simulink model "TosAeb"  (R2021a)
settings: Solver=FixedStepDiscrete  StartTime=0.0  StopTime=inf  FixedStep=0.01  RelTol=1e-3
undefined symbols (not defined in the file or in its folder): ACCModSts, AccSts1, AEB_ObjectMovingOrientation, AEB_ObjectMovingState, CN_BTCActive, CN_CurrentGearR, CN_CurrentGearR_DHT, CN_PTCActive, CN_VDCActive, CN_VSC_EspOff, CN_VSC_FCW_AEB_Error, CN_VSC_FCW_AEB_NoDispay, CN_VSC_FCW_AEB_PerfmDgdtn, CN_VSC_FCW_AEB_TempUnAvlbl, CN_VSC_VehStandstill, CNaccelAvoidMax, CNaccelAvoidMin, CNbrakePedalPress, CNcomfortStrLeftAccelMax, CNcomfortStrLeftAccelMin, CNcomfortStrRightAccelMax, CNcomfortStrRightAccelMin, CNDrvDoorOpen, CNDrvModeEco, CNDrvModeNorm, CNDrvModeOther, CNDrvModeSport, CNDrvSBRreminder, CNNull, CNobjInvalidID, CNPassDoorOpen, CNPassSBRreminder, CNPEBSAccelNegInf, CNPEBSAccelNull, CNPEBSTimeInf, CNPEBSTimeNull, CNSTOPMAX, CNSTOPNULL, CNsubjWidth, CNSwtOFF, CNSwtON, CNTTCMAX, CNTTCNULL, CNVehConfigAEBCycNone, CNVehConfigAEBNone, CNVehConfigAEBPedNone, CNVehConfigFCWNone, CNVehConfigJANone, CollisionSeverity, FALSE, FCWSnvty, JACycObjCalcInfoDefaultParams, JAPedObjCalcInfoDefaultParams, JAVehObjCalcInfoDefaultParams, latCycObjCalcInfoDefaultParams, latPedObjCalcInfoDefaultParams, longObjCalcInfoDefaultParams, ModSts, movingStatus, ObjectType ... (+1112)
libraries: simulink x3031, AEBSFunctionLib x50, Ta_Library x33, AEBSStateMachineLib x23, Mylibrary x12, simulink_extras x7, ObjSelect_lib x3, dspstat3 x2
(part 6 of 102)

== system TosAeb/AEB_Objsel/TA_Processor/AEB_ObjSelect/TargetInfoCheck/Each_Set ==
  TA_ObjSumInfo : Inport
  Bus Selector : BusSelector  OutputSignals=TA_ObjID,TA_ObjType,TA_ObjState,TA_ObjOrientation,TA_ObjfusType,TA_ObjLaneAssign,TA_ObjDx,TA_ObjDy,TA_ObjDxStd,TA_ObjDyStd,TA_ObjVx,TA_ObjVy,TA_ObjVxStd,TA_ObjVyStd,TA_ObjVxAbs,TA_ObjVyAbs,TA_ObjLength,TA_ObjWidth,TA_ObjHight,TA_ObjAx,TA_ObjAy,TA_ObjAxAbs,TA_ObjAyAbs,TA_ObjConfidence,TA_ObjCenterAngle,TA_ObjHeadAngle,TA_ObjHeadAngleStd,TA_ObjID
  Signal Conversion{,1..27} (28 alike) : SignalConversion  OverrideOpt=off
  TA_ObjID : Outport
  TA_ObjType : Outport  Port=2
  TA_ObjState : Outport  Port=3
  TA_ObjOrientation : Outport  Port=4
  TA_ObjfusType : Outport  Port=5
  TA_ObjLaneAssign : Outport  Port=6
  TA_ObjDx : Outport  Port=7
  TA_ObjDy : Outport  Port=8
  TA_ObjDxStd : Outport  Port=9
  TA_ObjDyStd : Outport  Port=10
  TA_ObjVx : Outport  Port=11
  TA_ObjVy : Outport  Port=12
  TA_ObjVxStd : Outport  Port=13
  TA_ObjVyStd : Outport  Port=14
  TA_ObjVxAbs : Outport  Port=15
  TA_ObjVyAbs : Outport  Port=16
  TA_ObjLength : Outport  Port=17
  TA_ObjWidth : Outport  Port=18
  TA_ObjHight : Outport  Port=19
  TA_ObjAx : Outport  Port=20
  TA_ObjAy : Outport  Port=21
  TA_ObjAxAbs : Outport  Port=22
  TA_ObjAyAbs : Outport  Port=23
  TA_ObjConfidence : Outport  Port=24
  TA_ObjCenterAngle : Outport  Port=25
  TA_ObjHeadAngle : Outport  Port=26
  TA_ObjHeadAngleStd : Outport  Port=27
  IDForeach : Outport  Port=28
  wires:
  TA_ObjSumInfo/1 -> Bus Selector/1
  Bus Selector/1 -> Signal Conversion/1  "<TA_ObjID>"
  Bus Selector/2 -> Signal Conversion1/1  "<TA_ObjType>"
  Bus Selector/3 -> Signal Conversion2/1  "<TA_ObjState>"
  Bus Selector/4 -> Signal Conversion3/1  "<TA_ObjOrientation>"
  Bus Selector/5 -> Signal Conversion4/1  "<TA_ObjfusType>"
  Bus Selector/6 -> Signal Conversion5/1  "<TA_ObjLaneAssign>"
  Bus Selector/7 -> Signal Conversion6/1  "<TA_ObjDx>"
  Bus Selector/8 -> Signal Conversion7/1  "<TA_ObjDy>"
  Bus Selector/9 -> Signal Conversion8/1  "<TA_ObjDxStd>"
  Bus Selector/10 -> Signal Conversion9/1  "<TA_ObjDyStd>"
  Bus Selector/11 -> Signal Conversion10/1  "<TA_ObjVx>"
  Bus Selector/12 -> Signal Conversion11/1  "<TA_ObjVy>"
  Bus Selector/13 -> Signal Conversion12/1  "<TA_ObjVxStd>"
  Bus Selector/14 -> Signal Conversion13/1  "<TA_ObjVyStd>"
  Bus Selector/15 -> Signal Conversion14/1  "<TA_ObjVxAbs>"
  Bus Selector/16 -> Signal Conversion15/1  "<TA_ObjVyAbs>"
  Bus Selector/17 -> Signal Conversion16/1  "<TA_ObjLength>"
  Bus Selector/18 -> Signal Conversion17/1  "<TA_ObjWidth>"
  Bus Selector/19 -> Signal Conversion18/1  "<TA_ObjHight>"
  Bus Selector/20 -> Signal Conversion19/1  "<TA_ObjAx>"
  Bus Selector/21 -> Signal Conversion20/1  "<TA_ObjAy>"
  Bus Selector/22 -> Signal Conversion21/1  "<TA_ObjAxAbs>"
  Bus Selector/23 -> Signal Conversion22/1  "<TA_ObjAyAbs>"
  Bus Selector/24 -> Signal Conversion23/1  "<TA_ObjConfidence>"
  Bus Selector/25 -> Signal Conversion24/1  "<TA_ObjCenterAngle>"
  Bus Selector/26 -> Signal Conversion25/1  "<TA_ObjHeadAngle>"
  Bus Selector/27 -> Signal Conversion26/1  "<TA_ObjHeadAngleStd>"
  Bus Selector/28 -> Signal Conversion27/1  "<TA_ObjID>"
  Signal Conversion/1 -> TA_ObjID/1
  Signal Conversion1/1 -> TA_ObjType/1
  Signal Conversion2/1 -> TA_ObjState/1
  Signal Conversion3/1 -> TA_ObjOrientation/1
  Signal Conversion4/1 -> TA_ObjfusType/1
  Signal Conversion5/1 -> TA_ObjLaneAssign/1
  Signal Conversion6/1 -> TA_ObjDx/1
  Signal Conversion7/1 -> TA_ObjDy/1
  Signal Conversion8/1 -> TA_ObjDxStd/1
  Signal Conversion9/1 -> TA_ObjDyStd/1
  Signal Conversion10/1 -> TA_ObjVx/1
  Signal Conversion11/1 -> TA_ObjVy/1
  Signal Conversion12/1 -> TA_ObjVxStd/1
  Signal Conversion13/1 -> TA_ObjVyStd/1
  Signal Conversion14/1 -> TA_ObjVxAbs/1
  Signal Conversion15/1 -> TA_ObjVyAbs/1
  Signal Conversion16/1 -> TA_ObjLength/1
  Signal Conversion17/1 -> TA_ObjWidth/1
  Signal Conversion18/1 -> TA_ObjHight/1
  Signal Conversion19/1 -> TA_ObjAx/1
  Signal Conversion20/1 -> TA_ObjAy/1
  Signal Conversion21/1 -> TA_ObjAxAbs/1
  Signal Conversion22/1 -> TA_ObjAyAbs/1
  Signal Conversion23/1 -> TA_ObjConfidence/1
  Signal Conversion24/1 -> TA_ObjCenterAngle/1
  Signal Conversion25/1 -> TA_ObjHeadAngle/1
  Signal Conversion26/1 -> TA_ObjHeadAngleStd/1
  Signal Conversion27/1 -> IDForeach/1

== system TosAeb/AEB_Objsel/TA_Processor/AEB_ObjSelect/TargetSelect/JA_ObjSelect ==
  TA_ObjInfo : Inport
  JAObjCollOut : Inport  Port=2
  Bus Selector : BusSelector  OutputSignals=TA_ObjID
  Bus Selector1 : BusSelector  OutputSignals=JA_ObjTcc,JA_ObjCollFlg
  MATLAB Function : MATLAB Function  TreatAsAtomicUnit=on
  JA_ObjSortNum : Outport
  JA_VaildID : Outport  Port=2
  wires:
  TA_ObjInfo/1 -> Bus Selector/1
  JAObjCollOut/1 -> Bus Selector1/1
  Bus Selector/1 -> MATLAB Function/1(ID)  "<TA_ObjID>"
  Bus Selector1/1 -> MATLAB Function/2(TTC)  "<JA_ObjTcc>"
  Bus Selector1/2 -> MATLAB Function/3(CllsnFlg)  "<JA_ObjCollFlg>"
  MATLAB Function/1(SortNum) -> JA_ObjSortNum/1
  MATLAB Function/2(VaildID) -> JA_VaildID/1

== system TosAeb/AEB_Objsel/TA_Processor/AEB_ObjSelect/TargetSelect/Lat_ObjSelect ==
  LatObj_Valid : Inport
  Lat_ObjIntersect : Inport  Port=2
  Lat_TTR : Inport  Port=3
  TA_ObjInfo : Inport  Port=4
  AEB_LatObj : Subsystem
  Lat_ValidNumber : Subsystem  TreatAsAtomicUnit=on
  Lat_ObjSortNum : Outport
  Lat_ObjID : Outport  Port=2
  wires:
  Lat_ValidNumber/1(Lat_ObjNum) -> AEB_LatObj/1(Lat_ObjNum)
  TA_ObjInfo/1 -> AEB_LatObj/3(TA_ObjInfo)
  LatObj_Valid/1 -> AEB_LatObj/2(LatObj_Valid), Lat_ValidNumber/1(LatObj_Valid)
  Lat_ObjIntersect/1 -> AEB_LatObj/4(Lat_ObjIntersect)
  Lat_TTR/1 -> AEB_LatObj/5(Lat_TTR)
  AEB_LatObj/1(Lat_ObjSortNum) -> Lat_ObjSortNum/1
  AEB_LatObj/2(Lat_ObjID) -> Lat_ObjID/1

== system TosAeb/AEB_Objsel/TA_Processor/AEB_ObjSelect/TargetSelect/Long_ObjSelect ==
  TA_ObjInfo : Inport
  VehSelfData : Inport  Port=2
  Boudingbox_Calculate : Inport  Port=3
  Obj_Coef : Inport  Port=4
  TTI : Inport  Port=5
  AccTgtIdn : Inport  Port=6
  Overlap_Rate : Inport  Port=7
  Obj_IDConfirm : Subsystem
  Long_ObjSortNum : Outport
  Long_ObjID : Outport  Port=2
  long_TTC : Outport  Port=3
  wires:
  VehSelfData/1 -> Obj_IDConfirm/2(VehSelfData)
  Obj_Coef/1 -> Obj_IDConfirm/4(Obj_Coef)
  TA_ObjInfo/1 -> Obj_IDConfirm/1(TA_ObjInfo)
  Boudingbox_Calculate/1 -> Obj_IDConfirm/3(Boudingbox_Calculate)
  TTI/1 -> Obj_IDConfirm/5(TTI)
  AccTgtIdn/1 -> Obj_IDConfirm/6(AccTgtIdn)
  Obj_IDConfirm/1(ValidTarget_ID) -> Long_ObjID/1
  Obj_IDConfirm/2(ID_num) -> Long_ObjSortNum/1
  Overlap_Rate/1 -> Obj_IDConfirm/7(Overlap_Rate)
  Obj_IDConfirm/3(long_TTC) -> long_TTC/1

== system TosAeb/AEB_Objsel/TA_Processor/PreProcessor/ExtractAccTargetIdIfAccActive/ConfirmAccId ==
  Acc_TaP0Id : Inport
  Constant : Constant  Value=uint8(0)  OutDataTypeStr=uint8
  Constant1 : Constant  Value=P_TA_AccTarCfmDlyInTaSlection
  Constant2 : Constant  Value=P_TA_AccTarCfmDlyEnadInTaSlection
  Constant4 : Constant  Value=P_TA_CallFrequencyInTaSlection
  Logical Operator : Logic  AllPortsSameDT=off  OutDataTypeStr=boolean
  OnDelay : Ta_Library/OnDelay  SystemSampleTime=-1  VariantActivationTime=update diagram  Latency=0  AutoFrameSizeCalculation=off
  Relational Operator : RelationalOperator  Operator===  OutDataTypeStr=boolean  RndMeth=Simplest
  Switch : Switch  Criteria=u2 ~= 0
  Unit Delay : UnitDelay  SampleTime=-1  HasFrameUpgradeWarning=on
  Confirmed_ AccP0Id : Outport
  wires:
  Constant2/1 -> Logical Operator/2
  Constant1/1 -> OnDelay/2
  Constant4/1 -> OnDelay/3
  Logical Operator/1 -> Switch/2
  Switch/1 -> Confirmed_ AccP0Id/1
  Constant/1 -> Switch/3
  OnDelay/1 -> Logical Operator/1  "AccIdHasBeenConstantForLongTime"
  Unit Delay/1 -> Relational Operator/2
  Relational Operator/1 -> OnDelay/1  "SameIdInLastSample"
  Acc_TaP0Id/1 -> Unit Delay/1, Relational Operator/1, Switch/1

== system TosAeb/AEB_Objsel/TA_Processor/PreProcessor/ExtractAccTargetIdIfAccActive/Enumerated Constant3 ==
  Constant : Constant  DialogController=Simulink.DDGSource  Value=AccSts1.Stop  OutDataTypeStr=Enum: AccSts1  LockScale=on
  Out : Outport
  wires:
  Constant/1 -> Out/1

== system TosAeb/AEB_Objsel/TA_Processor/PreProcessor/ExtractAccTargetIdIfAccActive/Enumerated Constant4 ==
  Constant : Constant  DialogController=Simulink.DDGSource  Value=AccSts1.StopTmp  OutDataTypeStr=Enum: AccSts1  LockScale=on
  Out : Outport
  wires:
  Constant/1 -> Out/1

== system TosAeb/AEB_Objsel/TA_Processor/PreProcessor/ExtractAccTargetIdIfAccActive/Enumerated Constant5 ==
  Constant : Constant  DialogController=Simulink.DDGSource  Value=AccSts1.Actv  OutDataTypeStr=Enum: AccSts1  LockScale=on
  Out : Outport
  wires:
  Constant/1 -> Out/1

== system TosAeb/AEB_Objsel/TA_Processor/PreProcessor/ObjectPrecessor/TAObjInfoCalc ==
  VehSelfIn : Inport
  Fus_TargetBus : Inport  Port=2
  Bus Selector : BusSelector  OutputSignals=Cam_ObsID_u8,Cam_ObsValid_u8,Cam_ObsAge_u16,Cam_ObsConfidence_u8,Cam_ObsMotionCategory_u8,Cam_ObsMotionStatus_u8,Cam_ObsMeasureStatus_u8,Cam_ObsClass_u8,Cam_ObsVhSubType_u8,Cam_ObsPedSubType_u8,Cam_ObsPedPos_u8,Cam_ObsPedOrientation_u8,Cam_ObsLaneAssign_u8,Cam_ObsLength_s,Cam_ObsWidth_s,Cam_ObsHeight_s,Cam_ObsCIPV_b,Cam_ObsMCP_b,Cam_ObsBlinker_u8,Cam_ObsBrkLgt_b,Cam_ObsCenterAngle_s,Cam_ObsPosType_u8,Cam_ObsPosX_s,Cam_ObsPosY_s,Cam_ObsVelX_s,Cam_ObsVelY_s,Cam_ObsAccX_s,Cam_ObsPosXSTD_s,Cam_ObsPosYSTD_s,Cam_ObsVelXSTD_s,Cam_ObsVelYSTD_s,Cam_ObsAccY_s,Cam_ObsAngle_s,Cam_ObsAngleSTD_s,Cam_ObsVelXAbs_s,Cam_ObsVelYAbs_s,Cam_ObsCornerPntX_s,Cam_ObsCutInDis_s,Cam_ObsCornerPntY_s,Cam_ObsCutInFlg_u8,Cam_ObsCutInSpd_s,Cam_ObsCutInSpdValid_b,Cam_ObsProNotOnRoad_b,Cam_ObsProCoverdByOthers_b,Cam_ObsProObserveJump_b,Cam_ObsProObserveSide_b,Cam_ObsProBtTapLevel1_b,Cam_ObsProBtTapLevel2_b,Cam_ObsProBtTapLevel3_b,Cam_ObsID_u8
  For Each Subsystem : Subsystem  TreatAsAtomicUnit=on
  Signal_Assignment : Subsystem
  TA_ObjInfo : Outport
  wires:
  For Each Subsystem/10(Fus_ObjQuality) -> Signal_Assignment/10(Fus_ObjQuality)
  For Each Subsystem/5(Fus_ObjLaneAssign) -> Signal_Assignment/5(Fus_ObjLaneAssign)
  For Each Subsystem/3(Fus_ObjState) -> Signal_Assignment/3(Fus_ObjState)
  For Each Subsystem/9(Fus_ObjVy) -> Signal_Assignment/9(Fus_ObjVy)
  For Each Subsystem/23(Fus_VelySTD) -> Signal_Assignment/23(Fus_VelySTD)
  For Each Subsystem/1(Fus_ObjID) -> Signal_Assignment/1(Fus_ObjID)
  For Each Subsystem/6(Fus_ObjDy) -> Signal_Assignment/6(Fus_ObjDy)
  For Each Subsystem/14(Fus_ObjVyAbs) -> Signal_Assignment/14(Fus_ObjVyAbs)
  For Each Subsystem/17(Fus_ObjLength) -> Signal_Assignment/17(Fus_ObjLength)
  For Each Subsystem/8(Fus_ObjVx) -> Signal_Assignment/8(Fus_ObjVx)
  For Each Subsystem/7(Fus_ObjDx) -> Signal_Assignment/7(Fus_ObjDx)
  For Each Subsystem/18(Fus_ObjWidth) -> Signal_Assignment/18(Fus_ObjWidth)
  For Each Subsystem/27(Fus_AyAbs) -> Signal_Assignment/27(Fus_AyAbs)
  For Each Subsystem/11(Fus_ObjAy) -> Signal_Assignment/11(Fus_ObjAy)
  For Each Subsystem/12(Fus_ObjAx) -> Signal_Assignment/12(Fus_ObjAx)
  For Each Subsystem/20(Fus_dxSTD) -> Signal_Assignment/20(Fus_dxSTD)
  For Each Subsystem/21(Fus_dySTD) -> Signal_Assignment/21(Fus_dySTD)
  Signal_Assignment/1(TA_ObjInfo) -> TA_ObjInfo/1
  For Each Subsystem/19(Fus_ObjHight) -> Signal_Assignment/19(Fus_ObjHight)
  For Each Subsystem/22(Fus_VelxSTD) -> Signal_Assignment/22(Fus_VelxSTD)
  For Each Subsystem/16(Fus_ObjType) -> Signal_Assignment/16(Fus_ObjType)
  For Each Subsystem/13(Fus_ObjVxAbs) -> Signal_Assignment/13(Fus_ObjVxAbs)
  For Each Subsystem/2(Fus_ObjOrientation) -> Signal_Assignment/2(Fus_ObjOrientation)
  For Each Subsystem/26(Fus_AxAbs) -> Signal_Assignment/26(Fus_AxAbs)
  For Each Subsystem/4(Fus_ObjfusType) -> Signal_Assignment/4(Fus_ObjfusType)
  For Each Subsystem/15(Fus_ObjHeadAngle) -> Signal_Assignment/15(Fus_ObjHeadAngle)
  VehSelfIn/1 -> For Each Subsystem/51(VehSelfIn)
  For Each Subsystem/25(Fus_CenterAngle) -> Signal_Assignment/25(Fus_CenterAngle)
  Fus_TargetBus/1 -> Bus Selector/1
  For Each Subsystem/24(Fus_HeadAngleSTD) -> Signal_Assignment/24(Fus_HeadAngleSTD)
  Bus Selector/1 -> For Each Subsystem/1(Cam_ObsID_u8)  "<Cam_ObsID_u8>"
  Bus Selector/2 -> For Each Subsystem/2(Cam_ObsValid_u8)  "<Cam_ObsValid_u8>"
  Bus Selector/3 -> For Each Subsystem/3(Cam_ObsAge_u16)  "<Cam_ObsAge_u16>"
  Bus Selector/4 -> For Each Subsystem/4(Cam_ObsConfidence_u8)  "<Cam_ObsConfidence_u8>"
  Bus Selector/5 -> For Each Subsystem/5(Cam_ObsMotionCategory_u8)  "<Cam_ObsMotionCategory_u8>"
  Bus Selector/6 -> For Each Subsystem/6(Cam_ObsMotionStatus_u8)  "<Cam_ObsMotionStatus_u8>"
  Bus Selector/7 -> For Each Subsystem/7(Cam_ObsMeasureStatus_u8)  "<Cam_ObsMeasureStatus_u8>"
  Bus Selector/8 -> For Each Subsystem/8(Cam_ObsClass_u8)  "<Cam_ObsClass_u8>"
  Bus Selector/9 -> For Each Subsystem/9(Cam_ObsVhSubType_u8)  "<Cam_ObsVhSubType_u8>"
  Bus Selector/10 -> For Each Subsystem/10(Cam_ObsPedSubType_u8)  "<Cam_ObsPedSubType_u8>"
  Bus Selector/11 -> For Each Subsystem/11(Cam_ObsPedPos_u8)  "<Cam_ObsPedPos_u8>"
  Bus Selector/12 -> For Each Subsystem/12(Cam_ObsPedOrientation_u8)  "<Cam_ObsPedOrientation_u8>"
  Bus Selector/13 -> For Each Subsystem/13(Cam_ObsLaneAssign_u8)  "<Cam_ObsLaneAssign_u8>"
  Bus Selector/14 -> For Each Subsystem/14(Cam_ObsLength_s)  "<Cam_ObsLength_s>"
  Bus Selector/15 -> For Each Subsystem/15(Cam_ObsWidth_s)  "<Cam_ObsWidth_s>"
  Bus Selector/16 -> For Each Subsystem/16(Cam_ObsHeight_s)  "<Cam_ObsHeight_s>"
  Bus Selector/17 -> For Each Subsystem/17(Cam_ObsCIPV_b)  "<Cam_ObsCIPV_b>"
  Bus Selector/18 -> For Each Subsystem/18(Cam_ObsMCP_b)  "<Cam_ObsMCP_b>"
  Bus Selector/19 -> For Each Subsystem/19(Cam_ObsBlinker_u8)  "<Cam_ObsBlinker_u8>"
  Bus Selector/20 -> For Each Subsystem/20(Cam_ObsBrkLgt_b)  "<Cam_ObsBrkLgt_b>"
  Bus Selector/21 -> For Each Subsystem/21(Cam_ObsCenterAngle_s)  "<Cam_ObsCenterAngle_s>"
  Bus Selector/22 -> For Each Subsystem/22(Cam_ObsPosType_u8)  "<Cam_ObsPosType_u8>"
  Bus Selector/23 -> For Each Subsystem/23(Cam_ObsPosX_s)  "<Cam_ObsPosX_s>"
  Bus Selector/24 -> For Each Subsystem/24(Cam_ObsPosY_s)  "<Cam_ObsPosY_s>"
  Bus Selector/25 -> For Each Subsystem/25(Cam_ObsVelX_s)  "<Cam_ObsVelX_s>"
  Bus Selector/26 -> For Each Subsystem/26(Cam_ObsVelY_s)  "<Cam_ObsVelY_s>"
  Bus Selector/27 -> For Each Subsystem/27(Cam_ObsAccX_s)  "<Cam_ObsAccX_s>"
  Bus Selector/28 -> For Each Subsystem/28(Cam_ObsPosXSTD_s)  "<Cam_ObsPosXSTD_s>"
  Bus Selector/29 -> For Each Subsystem/29(Cam_ObsPosYSTD_s)  "<Cam_ObsPosYSTD_s>"
  Bus Selector/30 -> For Each Subsystem/30(Cam_ObsVelXSTD_s)  "<Cam_ObsVelXSTD_s>"
  Bus Selector/31 -> For Each Subsystem/31(Cam_ObsVelYSTD_s)  "<Cam_ObsVelYSTD_s>"
  Bus Selector/32 -> For Each Subsystem/32(Cam_ObsAccY_s)  "<Cam_ObsAccY_s>"
  Bus Selector/33 -> For Each Subsystem/33(Cam_ObsAngle_s)  "<Cam_ObsAngle_s>"
  Bus Selector/34 -> For Each Subsystem/34(Cam_ObsAngleSTD_s)  "<Cam_ObsAngleSTD_s>"
  Bus Selector/35 -> For Each Subsystem/35(Cam_ObsVelXAbs_s)  "<Cam_ObsVelXAbs_s>"
  Bus Selector/36 -> For Each Subsystem/36(Cam_ObsVelYAbs_s)  "<Cam_ObsVelYAbs_s>"
  Bus Selector/37 -> For Each Subsystem/37(Cam_ObsCornerPntX_s)  "<Cam_ObsCornerPntX_s>"
  Bus Selector/38 -> For Each Subsystem/38(Cam_ObsCutInDis_s)  "<Cam_ObsCutInDis_s>"
  Bus Selector/39 -> For Each Subsystem/39(Cam_ObsCornerPntY_s)  "<Cam_ObsCornerPntY_s>"
  Bus Selector/40 -> For Each Subsystem/40(Cam_ObsCutInFlg_u8)  "<Cam_ObsCutInFlg_u8>"
  Bus Selector/41 -> For Each Subsystem/41(Cam_ObsCutInSpd_s)  "<Cam_ObsCutInSpd_s>"
  Bus Selector/42 -> For Each Subsystem/42(Cam_ObsCutInSpdValid_b)  "<Cam_ObsCutInSpdValid_b>"
  Bus Selector/43 -> For Each Subsystem/43(Cam_ObsProNotOnRoad_b)  "<Cam_ObsProNotOnRoad_b>"
  Bus Selector/44 -> For Each Subsystem/44(Cam_ObsProCoverdByOthers_b)  "<Cam_ObsProCoverdByOthers_b>"
  Bus Selector/45 -> For Each Subsystem/45(Cam_ObsProObserveJump_b)  "<Cam_ObsProObserveJump_b>"
  Bus Selector/46 -> For Each Subsystem/46(Cam_ObsProObserveSide_b)  "<Cam_ObsProObserveSide_b>"
  Bus Selector/47 -> For Each Subsystem/47(Cam_ObsProBtTapLevel1_b)  "<Cam_ObsProBtTapLevel1_b>"
  Bus Selector/48 -> For Each Subsystem/48(Cam_ObsProBtTapLevel2_b)  "<Cam_ObsProBtTapLevel2_b>"
  Bus Selector/49 -> For Each Subsystem/49(Cam_ObsProBtTapLevel3_b)  "<Cam_ObsProBtTapLevel3_b>"
  Bus Selector/50 -> For Each Subsystem/50(IDForEach)  "<Cam_ObsID_u8>"

== system TosAeb/AEB_Objsel/TA_Processor/PreProcessor/VehiclePreProcessor/CalculateCurvature ==
  note: [8 10]
  note: [0 1]
  note: 自车纵向车速低于8m/s时，使用YawRate来计算自车运动曲率
      | 自车纵向车速高于10m/s时，使用二自由度自行车模型来计算自车运动曲率
      | 自车纵向车速在8-10m/s范围内时，采用插值取权重的方法来计算自车运动曲率
  VehSelf : Inport
  Bus Selector1 : BusSelector  OutputSignals=ABS_VehicleSpeed,SAS_SteeringWheelAngle
  Bus Selector2 : BusSelector  OutputSignals=SAS_SteeringWheelSpeed
  Bus Selector4 : BusSelector  OutputSignals=YawRate
  Constant : Constant  Value=single(1)
  Constant1 : Constant  Value=single(0)
  LookUpWeightFactor : Lookup_n-D  NumberOfTableDimensions=1  BreakpointsForDimension1=P_DM_DrvVLgtForCrvtTable  Table=P_DM_DrvCrvtCalcWeightFactor  ExtrapMethod=Clip  RemoveProtectionInput=on  UseLastTableValue=on  InternalRulePriority=Speed  RndMeth=Simplest  InputSameDT=on
      settings: AttributesFormatString=|
      | UseLastTableValue=%<UseLastTableValue>
      | RemoveProtectionInput=%<RemoveProtectionInput>
      | RndMeth=%<RndMeth>
  LowPassFilter : Subsystem
  SafeDivide : Subsystem  TreatAsAtomicUnit=on  mask(Safe divide){DataType=single, DataTypeMax=0, DataTypeMin=0}
      mask prompts: DataType "Data type:"
      mask help:
      | This block offers division protected from division by zero.
      |
      | When division by zero is detected, a very large number will be returned with the same sign as the numerator.
      |
      | In the case zero divided by zero, the output has been defined as zero.
      |
      | Both floating-point numbers and integers are supported.
      mask init:
      | % Author: Jakob Andersson (jande573)
      | 
      | % Test if the selected data type is a floating point type or an integer
      | % The supported data types is selected on the parameters pane
      | if(strcmp(DataType,'double')||strcmp(DataType,'single'))
      |     % Find the max and min values of the selected data type
      |     DataTypeMax=fix(sqrt(realmax(DataType))); % In order to avoid errors when operating on the output
      |     DataTypeMin=-fix(sqrt(realmax(DataType))); % In order to avoid errors when operating on the output
      | else
      |     % Find the max and min values of the selected data type
      |     DataTypeMax=intmax(DataType);
      |     DataTypeMin=-intmax(DataType);
      | end
      settings: RTWSystemCode=Inline
      mask Help:
      | This block offers division protected from division by zero.
      | 
      | When division by zero is detected, a very large number will be returned with the same sign as the numerator.
      | 
      | In the case zero divided by zero, the output has been defined as zero.
      | 
      | Both floating-point numbers and integers are supported.
      mask Display:
      | % Author: Jakob Andersson (jande573)
      | 
      | % Display the allowed data type
      | disp(DataType);
      | 
      | % Display the port symbols
      | port_label('input',1,'\bullet','texmode','on');
      | port_label('input',2,'\div','texmode','on');
  SafeDivide1 : Subsystem  TreatAsAtomicUnit=on  mask(Safe divide){DataType=single, DataTypeMax=0, DataTypeMin=0}
      mask prompts: DataType "Data type:"
      mask help:
      | This block offers division protected from division by zero.
      |
      | When division by zero is detected, a very large number will be returned with the same sign as the numerator.
      |
      | In the case zero divided by zero, the output has been defined as zero.
      |
      | Both floating-point numbers and integers are supported.
      mask init: same as TosAeb/AEB_Objsel/TA_Processor/PreProcessor/VehiclePreProcessor/CalculateCurvature/SafeDivide
      settings: RTWSystemCode=Inline
      mask Help:
      | This block offers division protected from division by zero.
      | 
      | When division by zero is detected, a very large number will be returned with the same sign as the numerator.
      | 
      | In the case zero divided by zero, the output has been defined as zero.
      | 
      | Both floating-point numbers and integers are supported.
      mask Display:
      | % Author: Jakob Andersson (jande573)
      | 
      | % Display the allowed data type
      | disp(DataType);
      | 
      | % Display the port symbols
      | port_label('input',1,'\bullet','texmode','on');
      | port_label('input',2,'\div','texmode','on');
  Saturation1 : Saturate  UpperLimit=single(0.1)  LowerLimit=single(-0.1)
      settings: AttributesFormatString=|
      | Limits:
      | [%<LowerLimit>, %<UpperLimit>]
  Saturation2 : Saturate  UpperLimit=single(0.1)  LowerLimit=single(-0.1)
      settings: AttributesFormatString=|
      | Limits:
      | [%<LowerLimit>, %<UpperLimit>]
  StaticBicycleModelC0 : Subsystem
  StaticBicycleModelC1 : Subsystem
  Weighting : Subsystem  mask(Blending){}
      mask help:
      | Mixes input X1 with input X2 using the weight factor, w.
      | Y = X1*w + X2*(1-w).
      | If w >= 1, Y = X1.
      | If w <= 0, Y = X2.
  Weighting1 : Subsystem  mask(Blending){}
      mask help:
      | Mixes input X1 with input X2 using the weight factor, w.
      | Y = X1*w + X2*(1-w).
      | If w >= 1, Y = X1.
      | If w <= 0, Y = X2.
  Curvature : Outport
  CurvatureRate : Outport  Port=2
  CurvatureRadius : Outport  Port=3
  wires:
  Saturation2/1 -> CurvatureRate/1  "CurvatureRate"
  Saturation1/1 -> Curvature/1, SafeDivide1/2(Denominator)  "Curvature"
  Weighting1/1(Y) -> Saturation2/1
  [Weight] -> Weighting1/2(WeightFactor)
  Constant1/1 -> Weighting1/1(X1)
  LowPassFilter/1(PinionAngleRate) -> StaticBicycleModelC1/2(PinionSteerAgRate)
  SafeDivide1/1(Quotient) -> CurvatureRadius/1  "CurvatureRadius"
  Constant/1 -> SafeDivide1/1(Numerator)
  Bus Selector1/1 -> LookUpWeightFactor/1, SafeDivide/2(Denominator), StaticBicycleModelC0/1(VLgt), [VLgt]  "<ABS_VehicleSpeed>"
  StaticBicycleModelC0/1(CurvatureRear) -> Weighting/3(X2)  "PinionAngleBasedCurvature"
  Bus Selector4/1 -> SafeDivide/1(Numerator)  "<YawRate>"
  SafeDivide/1(Quotient) -> Weighting/1(X1)  "YawRateBasedCurvature"
  LookUpWeightFactor/1 -> Weighting/2(WeightFactor), [Weight]  "WeightFactor"
  Weighting/1(Y) -> Saturation1/1  "Curvature"
  StaticBicycleModelC1/1(CurvatureRear) -> Weighting1/3(X2)  "PinionAngleBasedCurvatureRate"
  [VLgt] -> StaticBicycleModelC1/1(VLgt)
  VehSelf/1 -> Bus Selector2/1, Bus Selector1/1, Bus Selector4/1
  Bus Selector1/2 -> StaticBicycleModelC0/2(PinionSteerAg)  "<SAS_SteeringWheelAngle>"
  Bus Selector2/1 -> LowPassFilter/1(PinionAngleRate_in)  "<SAS_SteeringWheelSpeed>"

== system TosAeb/AEB_Objsel/TA_Processor/PreProcessor/VehiclePreProcessor/LongitudinalAccelerationWithoutCMbB ==
  note: CMbB激活下发DriverAcceleration
      |           未激活下发ALgt
  VehSelf : Inport
  ActtnDataFromCMbB : Inport  Port=2
  Bus Selector1 : BusSelector  OutputSignals=ABS_MasterCylinderPressure
  Bus Selector2 : BusSelector  OutputSignals=AX1
  Constant : Constant  OutDataTypeStr=boolean
  Relop_1 : RelationalOperator  Operator===  OutDataTypeStr=boolean  RndMeth=Nearest
  Switch : Switch  Criteria=u2 ~= 0
  Unary Minus : UnaryMinus
  DrvrALgt : Outport
  wires:
  Constant/1 -> Relop_1/2
  Relop_1/1 -> Switch/2  "CMbBActive"
  Switch/1 -> DrvrALgt/1  "DrvrALgt"
  ActtnDataFromCMbB/1 -> Relop_1/1
  VehSelf/1 -> Bus Selector2/1, Bus Selector1/1
  Bus Selector1/1 -> Switch/3  "<ABS_MasterCylinderPressure>"
  Unary Minus/1 -> Switch/1  "DriverAcceleration"
  Bus Selector2/1 -> Unary Minus/1  "<AX1>"

== system TosAeb/AEB_Objsel/TA_Processor/PreProcessor/VehiclePreProcessor/PathEstimn_Calculate ==
  Veh_LgtSpd_mps : Inport
  Veh_LgtAccel_mps2 : Inport  Port=2
  Curvature : Inport  Port=3
  CurvatureRate : Inport  Port=4
  Bus Creator : BusCreator  Inputs=2
  For Iterator PahEstimn : Subsystem  TreatAsAtomicUnit=on
  PahEstimn : Outport
  wires:
  For Iterator PahEstimn/1(PahEstimn) -> PahEstimn/1
  Bus Creator/1 -> For Iterator PahEstimn/2(VehSelf)
  Veh_LgtAccel_mps2/1 -> Bus Creator/2  "Veh_LgtAccel_mps2"
  Veh_LgtSpd_mps/1 -> Bus Creator/1  "Veh_LgtSpd_mps"
  Curvature/1 -> For Iterator PahEstimn/1(Curvature)
  CurvatureRate/1 -> For Iterator PahEstimn/3(CurvatureRate)

== system TosAeb/AEB_Objsel/TA_Processor/TA_Check/TA_LatCheck/For Iterator Subsystem ==
  Lat_ObjSortNum : Inport
  Lat_TTC : Inport  Port=2
  Assignment : Assignment  DiagnosticForDimensions=Warning  IndexOptions=Index vector (port)  Indices=1  OutputSizes=1
  Compare To Constant : simulink/Logic and Bit Operations/Compare To Constant  relop===  const=0  OutDataTypeStr=boolean
  Constant : Constant  Value=zeros(10,1)  OutDataTypeStr=single
  Constant1 : Constant  Value=100  OutDataTypeStr=single
  Constant2 : Constant  OutDataTypeStr=uint8
  For Iterator : ForIterator  IterationLimit=10
  Index Vector : MultiPortSwitch  Inputs=1
  Index Vector1 : MultiPortSwitch  Inputs=1
  Switch : Switch  Criteria=u2 > Threshold
  Switch1 : Switch  Criteria=u2 > Threshold
  ttc : Outport
  wires:
  Constant/1 -> Assignment/1
  Assignment/1 -> ttc/1
  For Iterator/1 -> [Index]
  [Index] -> Assignment/3
  [Index] -> Index Vector1/1
  Lat_ObjSortNum/1 -> Index Vector1/2
  Index Vector1/1 -> Switch1/3, Compare To Constant/1
  Compare To Constant/1 -> Switch1/2, Switch/2
  Constant1/1 -> Switch/1
  Constant2/1 -> Switch1/1
  Switch1/1 -> Index Vector/1
  Lat_TTC/1 -> Index Vector/2
  Switch/1 -> Assignment/2
  Index Vector/1 -> Switch/3

== system TosAeb/ELK_Objsel/Target_Selection/If Action Subsystem/ELK_TargetSelection/If Action Subsystem ==
  Action Port : ActionPort  ActionPortLabel=else
  Constant : Constant  Value=0  OutDataTypeStr=boolean
  Out : Outport
  wires:
  Constant/1 -> Out/1

== system TosAeb/ELK_Objsel/Target_Selection/If Action Subsystem/ELK_TargetSelection/If Action Subsystem1 ==
  Action Port : ActionPort  ActionPortLabel=else
  Constant : Constant  Value=0  OutDataTypeStr=boolean
  Out1 : Outport
  wires:
  Constant/1 -> Out1/1

== system TosAeb/ELK_Objsel/Target_Selection/If Action Subsystem/ELK_TargetSelection/If Action Subsystem4 ==
  Action Port : ActionPort  ActionPortLabel=else
  Constant : Constant  Value=0  OutDataTypeStr=boolean
  Out : Outport
  wires:
  Constant/1 -> Out/1

== system TosAeb/ELK_Objsel/Target_Selection/If Action Subsystem/ELK_TargetSelection/If Action Subsystem5 ==
  Action Port : ActionPort  ActionPortLabel=else
  Constant : Constant  Value=0  OutDataTypeStr=boolean
  Out1 : Outport
  wires:
  Constant/1 -> Out1/1

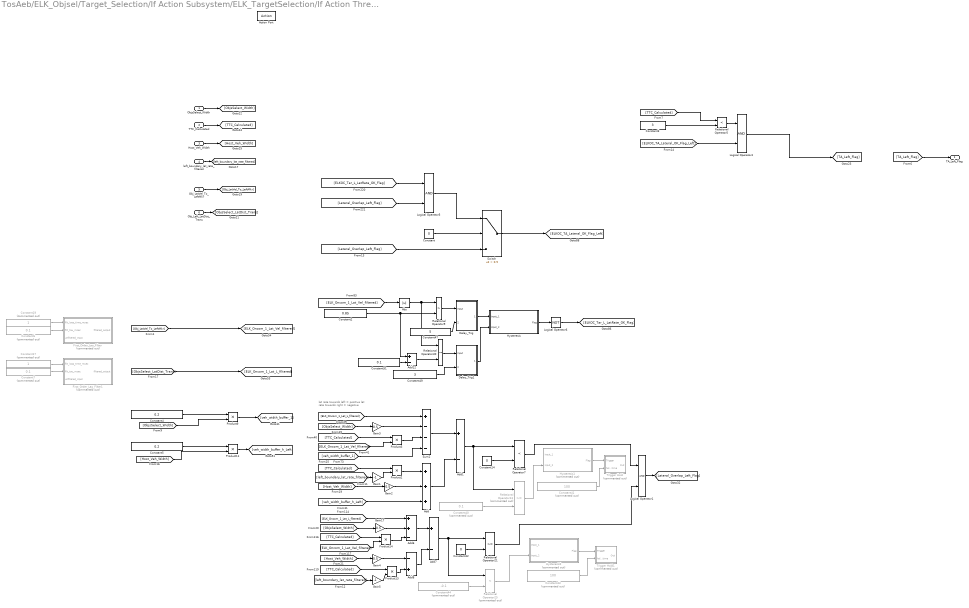
== system TosAeb/ELK_Objsel/Target_Selection/If Action Subsystem/ELK_TargetSelection/If Action Threat_Assessment_Left ==
  note: lat rate towards left = positive
      | lat rate towards right = negative
  ObjsSelect_Width : Inport
  TTC_Calculated : Inport  Port=2
  Host_Veh_Width : Inport  Port=3
  left_boundary_lat_rate_filtered : Inport  Port=4
  Obj_LatVel_To_LeftMkr : Inport  Port=5
  Obj_Left_LatDist_Trans : Inport  Port=6
  Action Port : ActionPort  ActionPortLabel=if(u1 ~= 0)
  Abs : Abs  Description=_GWMH9P4.ELK.000_40_
  Add : Sum  Description=_GWMH9P5.ELK.000_14_  Inputs=+++
  Add1 : Sum  Description=_GWMH9P5.ELK.000_15_  Inputs=+-
  Add12 : Sum  Description=_GWMH9P5.ELK.000_18_  Inputs=+-
  Add6 : Sum  Description=_GWMH9P5.ELK.000_22_  Inputs=++-
  Add7 : Sum  Description=_GWMH9P5.ELK.000_23_  Inputs=+-
  Add8 : Sum  Description=_GWMH9P5.ELK.000_24_  Inputs=-+
  Constant : Constant  Value=0
  Constant14 : Constant  Description=_GWMH9P4.ELK.000_42_  Value=0
  Constant17 : Constant  Description=_GWMH9P5.ELK.000_26_  Commented=on
  Constant19 : Constant  Description=_GWMH9P5.ELK.000_27_  Commented=on
  Constant2 : Constant  Description=_GWMH9P5.ELK.000_52_  Value=0.85
  Constant3 : Constant  Description=_GWMH9P5.ELK.000_38_  Value=3
  Constant4 : Constant  Description=_GWMH9P5.ELK.000_45_  Value=0.2
  Constant40 : Constant  Description=_GWMH9P5.ELK.000_41_  Commented=on  Value=0.1  mask(TL_Constant){data={'date',738384.81607121532}}
      settings: CopyFcn=if exist('tl_reset_reviewflag','file')==2,tl_reset_reviewflag(gcbh);end  DeleteFcn=if exist('delete_tlblock','file')==2,delete_tlblock(gcbh);end  NameChangeFcn=if exist('rename_tlblock','file')==2,rename_tlblock(gcbh);end  OpenFcn=if exist('tl_open_blockdlg','file')==2,tl_open_blockdlg(gcbh);else,open_system(gcbh,'parameter');end
      mask Help: eval(['if exist(''tl_online_help'',''file'') == 2, tl_online_help(''Topic'',''TL_Constant''),else, web([''jar:file:///'',matlabroot,''/help/toolbox/simulink/help.jar!/slref/' lower(get_param(gcbh,'Blocktype')) '.html''], ''-helpbrowser''),end'])
      mask Display: plot([-0.1, 1.05, NaN, -0.1, 1.05],[.01, .01, NaN, .99, .99]);
  Constant41 : Constant  Description=_GWMH9P5.ELK.000_42_  Commented=on  Value=100  mask(TL_Constant){data={'date',738384.81607467588}}
      settings: CopyFcn=if exist('tl_reset_reviewflag','file')==2,tl_reset_reviewflag(gcbh);end  DeleteFcn=if exist('delete_tlblock','file')==2,delete_tlblock(gcbh);end  NameChangeFcn=if exist('rename_tlblock','file')==2,rename_tlblock(gcbh);end  OpenFcn=if exist('tl_open_blockdlg','file')==2,tl_open_blockdlg(gcbh);else,open_system(gcbh,'parameter');end
      mask Help: eval(['if exist(''tl_online_help'',''file'') == 2, tl_online_help(''Topic'',''TL_Constant''),else, web([''jar:file:///'',matlabroot,''/help/toolbox/simulink/help.jar!/slref/' lower(get_param(gcbh,'Blocktype')) '.html''], ''-helpbrowser''),end'])
      mask Display: plot([-0.1, 1.05, NaN, -0.1, 1.05],[.01, .01, NaN, .99, .99]);
  Constant42 : Constant  Description=_GWMH9P5.ELK.000_43_  Value=0
  Constant43 : Constant  Description=_GWMH9P5.ELK.000_44_  Commented=on  Value=100  mask(TL_Constant){data={'date',738384.81608211808}}
      settings: CopyFcn=if exist('tl_reset_reviewflag','file')==2,tl_reset_reviewflag(gcbh);end  DeleteFcn=if exist('delete_tlblock','file')==2,delete_tlblock(gcbh);end  NameChangeFcn=if exist('rename_tlblock','file')==2,rename_tlblock(gcbh);end  OpenFcn=if exist('tl_open_blockdlg','file')==2,tl_open_blockdlg(gcbh);else,open_system(gcbh,'parameter');end
      mask Help: eval(['if exist(''tl_online_help'',''file'') == 2, tl_online_help(''Topic'',''TL_Constant''),else, web([''jar:file:///'',matlabroot,''/help/toolbox/simulink/help.jar!/slref/' lower(get_param(gcbh,'Blocktype')) '.html''], ''-helpbrowser''),end'])
      mask Display: plot([-0.1, 1.05, NaN, -0.1, 1.05],[.01, .01, NaN, .99, .99]);
  Constant44 : Constant  Description=_GWMH9P5.ELK.000_45_  Commented=on  Value=-0.1  mask(TL_Constant){data={'date',738384.81608572917}}
      settings: CopyFcn=if exist('tl_reset_reviewflag','file')==2,tl_reset_reviewflag(gcbh);end  DeleteFcn=if exist('delete_tlblock','file')==2,delete_tlblock(gcbh);end  NameChangeFcn=if exist('rename_tlblock','file')==2,rename_tlblock(gcbh);end  OpenFcn=if exist('tl_open_blockdlg','file')==2,tl_open_blockdlg(gcbh);else,open_system(gcbh,'parameter');end
      mask Help: eval(['if exist(''tl_online_help'',''file'') == 2, tl_online_help(''Topic'',''TL_Constant''),else, web([''jar:file:///'',matlabroot,''/help/toolbox/simulink/help.jar!/slref/' lower(get_param(gcbh,'Blocktype')) '.html''], ''-helpbrowser''),end'])
      mask Display: plot([-0.1, 1.05, NaN, -0.1, 1.05],[.01, .01, NaN, .99, .99]);
  Constant47 : Constant  Description=_GWMH9P5.ELK.000_48_  Value=5
  Constant49 : Constant  Description=_GWMH9P5.ELK.000_50_  Value=3
  Constant5 : Constant  Description=_GWMH9P5.ELK.000_45_  Value=0.2
  Constant51 : Constant  Description=_GWMH9P5.ELK.000_52_  Value=0.1
  Constant6 : Constant  Description=_GWMH9P5.ELK.000_27_  Commented=on  Value=0.1
  Constant7 : Constant  Description=_GWMH9P5.ELK.000_27_  Commented=on  Value=0.1
  Delay_Trig : Subsystem
  Delay_Trig1 : Subsystem
  First_Order_Lag_Filter : Subsystem  Commented=on
  First_Order_Lag_Filter1 : Subsystem  Commented=on
  Gain1 : Gain  Description=_GWMH9P4.ELK.000_64_  Gain=-1
  Gain17 : Gain  Description=_GWMH9P5.ELK.000_80_  Gain=0.5
  Gain2 : Gain  Description=_GWMH9P4.ELK.000_64_  Gain=0.5
  Gain3 : Gain  Description=_GWMH9P4.ELK.000_64_  Gain=0.5
  Gain4 : Gain  Description=_GWMH9P4.ELK.000_64_  Gain=0.5
  Gain5 : Gain  Description=_GWMH9P4.ELK.000_64_  Gain=-1
  Hysteresis : Stateflow chart  TreatAsAtomicUnit=on
  Hysteresis1 : Stateflow chart  Commented=on  TreatAsAtomicUnit=on
  Hysteresis2 : Stateflow chart  Commented=on  TreatAsAtomicUnit=on
  Logical Operator1 : Logic  Description=_GWMH9P5.ELK.000_86_  AllPortsSameDT=off  OutDataTypeStr=boolean
  Logical Operator4 : Logic  Description=_GWMH9P4.ELK.000_68_  AllPortsSameDT=off  OutDataTypeStr=boolean
  Logical Operator5 : Logic  Description=_GWMH9P4.ELK.000_68_  AllPortsSameDT=off  OutDataTypeStr=boolean
  Logical Operator6 : Logic  Description=_GWMH9P5.ELK.000_88_  Operator=NOT  Inputs=1  AllPortsSameDT=off  OutDataTypeStr=boolean
  Product1 : Product  Description=_GWMH9P4.ELK.000_71_  RndMeth=Zero
  Product11 : Product  Description=_GWMH9P5.ELK.000_91_
  Product13 : Product  Description=_GWMH9P5.ELK.000_93_  RndMeth=Zero
  Product14 : Product  Description=_GWMH9P5.ELK.000_94_  RndMeth=Zero
  Product3 : Product  Description=_GWMH9P4.ELK.000_73_  RndMeth=Zero
  Product9 : Product  Description=_GWMH9P5.ELK.000_102_
  Relational Operator13 : RelationalOperator  Description=_GWMH9P5.ELK.000_106_  Commented=on  OutDataTypeStr=boolean  RndMeth=Nearest  mask(TL_RelationalOperator){data={'date',738384.81682939816}}
      settings: DeleteFcn=if exist('delete_tlblock','file')==2,delete_tlblock(gcbh);end  NameChangeFcn=if exist('rename_tlblock','file')==2,rename_tlblock(gcbh);end  OpenFcn=if exist('tl_open_blockdlg','file')==2,tl_open_blockdlg(gcbh);else,open_system(gcbh,'parameter');end
      mask Help: eval(['if exist(''tl_online_help'',''file'') == 2, tl_online_help(''Topic'',''TL_RelationalOperator''),else, web([''jar:file:///'',matlabroot,''/help/toolbox/simulink/help.jar!/slref/' lower(get_param(gcbh,'Blocktype')) '.html''], ''-helpbrowser''),end'])
      mask Display: plot([-0.1, 1.05, NaN, -0.1, 1.05],[.01, .01, NaN, .99, .99]);
  Relational Operator21 : RelationalOperator  Description=_GWMH9P5.ELK.000_108_  OutDataTypeStr=boolean  RndMeth=Nearest
  Relational Operator25 : RelationalOperator  Description=_GWMH9P5.ELK.000_109_  Commented=on  Operator=<  OutDataTypeStr=boolean  RndMeth=Nearest  mask(TL_RelationalOperator){data={'date',738384.8168361343}}
      settings: DeleteFcn=if exist('delete_tlblock','file')==2,delete_tlblock(gcbh);end  NameChangeFcn=if exist('rename_tlblock','file')==2,rename_tlblock(gcbh);end  OpenFcn=if exist('tl_open_blockdlg','file')==2,tl_open_blockdlg(gcbh);else,open_system(gcbh,'parameter');end
      mask Help: eval(['if exist(''tl_online_help'',''file'') == 2, tl_online_help(''Topic'',''TL_RelationalOperator''),else, web([''jar:file:///'',matlabroot,''/help/toolbox/simulink/help.jar!/slref/' lower(get_param(gcbh,'Blocktype')) '.html''], ''-helpbrowser''),end'])
      mask Display: plot([-0.1, 1.05, NaN, -0.1, 1.05],[.01, .01, NaN, .99, .99]);
  Relational Operator30 : RelationalOperator  Description=_GWMH9P5.ELK.000_112_  Operator=<=  OutDataTypeStr=boolean  RndMeth=Nearest
  Relational Operator5 : RelationalOperator  Description=_GWMH9P3.ELK.000_91_  Operator=<  OutDataTypeStr=boolean  RndMeth=Nearest
  Relational Operator7 : RelationalOperator  Description=_GWMH9P4.ELK.000_81_  Operator=<  OutDataTypeStr=boolean  RndMeth=Nearest
  Relational Operator9 : RelationalOperator  Description=_GWMH9P5.ELK.000_114_  Operator=>  OutDataTypeStr=boolean  RndMeth=Nearest
  Sum2 : Sum  Description=_GWMH9P4.ELK.000_83_  Inputs=+---
  Switch : Switch  Criteria=u2 > Threshold  Threshold=0.5
  Trigger Hold : Subsystem  Commented=on
  Trigger Hold1 : Subsystem  Commented=on
  TA_Left_Flag : Outport
  wires:
  Logical Operator4/1 -> [TA_Left_Flag]
  Constant3/1 -> Relational Operator5/2
  [ELKOC_TA_Lateral_OK_Flag_Left] -> Logical Operator4/2
  [TTC_Calculated] -> Relational Operator5/1
  Relational Operator5/1 -> Logical Operator4/1
  [ELKOC_Tar_L_LatRate_OK_Flag] -> Logical Operator5/1
  [Lateral_Overlap_Left_Flag] -> Logical Operator5/2
  [TA_Left_Flag] -> TA_Left_Flag/1
  ObjsSelect_Width/1 -> [ObjsSelect_Width]
  TTC_Calculated/1 -> [TTC_Calculated]
  Host_Veh_Width/1 -> [Host_Veh_Width]
  left_boundary_lat_rate_filtered/1 -> [left_boundary_lat_rate_filtered]
  Obj_LatVel_To_LeftMkr/1 -> [Obj_LatVel_To_LeftMkr]
  Hysteresis/1(Flag) -> Logical Operator6/1
  Relational Operator9/1 -> Delay_Trig/1(Input)
  Constant51/1 -> Add12/2
  Add12/1 -> Relational Operator30/2
  Abs/1 -> Relational Operator9/1, Relational Operator30/1
  Constant49/1 -> Delay_Trig1/2(Delay_Cnt)
  Delay_Trig1/1(Output) -> Hysteresis/2(Input_2)
  Relational Operator30/1 -> Delay_Trig1/1(Input)
  Constant47/1 -> Delay_Trig/2(Delay_Cnt)
  Constant2/1 -> Relational Operator9/2, Add12/1
  [ELK_Oncom_1_Lat_Vel_filtered] -> Abs/1
  [Obj_LatVel_To_LeftMkr] -> [ELK_Oncom_1_Lat_Vel_filtered]
  Constant43/1 -> Trigger Hold1/2(hold_time)
  Hysteresis2/1(Flag) -> Trigger Hold1/1(Trigger)
  Relational Operator25/1 -> Hysteresis2/2(Input_2)
  Relational Operator21/1 -> Logical Operator1/2
  Constant44/1 -> Relational Operator25/2
  Add8/1 -> Add7/2
  Gain4/1 -> Add8/1
  Product13/1 -> Add8/2
  [ELK_Oncom_1_Lat_L_filtered] -> Add6/1
  Constant42/1 -> Relational Operator21/2
  Add7/1 -> Relational Operator21/1, Relational Operator25/1
  [left_boundary_lat_rate_filtered] -> Gain5/1
  [TTC_Calculated] -> Product13/1
  Add6/1 -> Add7/1
  Product14/1 -> Add6/3
  [ELK_Oncom_1_Lat_Vel_filtered] -> Product14/2
  [TTC_Calculated] -> Product14/1
  Gain17/1 -> Add6/2
  [ObjsSelect_Width] -> Gain17/1
  Constant41/1 -> Trigger Hold/2(hold_time)
  Relational Operator13/1 -> Hysteresis1/2(Input_2)
  Constant40/1 -> Relational Operator13/2
  Relational Operator7/1 -> Logical Operator1/1
  Hysteresis1/1(Flag) -> Trigger Hold/1(Trigger)
  Logical Operator1/1 -> [Lateral_Overlap_Left_Flag]
  Add1/1 -> Relational Operator7/1, Relational Operator13/1
  Add/1 -> Add1/2
  Sum2/1 -> Add1/1
  [veh_width_buffer_h_Left] -> Add/3
  [Host_Veh_Width] -> Gain2/1
  Product1/1 -> Add/1
  [veh_width_buffer_1] -> Sum2/4
  Product3/1 -> Sum2/3
  Gain3/1 -> Sum2/2
  [ELK_Oncom_1_Lat_L_filtered] -> Sum2/1
  [ELK_Oncom_1_Lat_Vel_filtered] -> Product3/2
  [TTC_Calculated] -> Product3/1
  Constant14/1 -> Relational Operator7/2
  [left_boundary_lat_rate_filtered] -> Gain1/1
  [ObjsSelect_Width] -> Gain3/1
  [TTC_Calculated] -> Product1/1
  [ObjsSelect_LatDist_Trans] -> [ELK_Oncom_1_Lat_L_filtered]
  [ObjsSelect_Width] -> Product9/2
  Constant4/1 -> Product9/1
  Product9/1 -> [veh_width_buffer_1]
  Product11/1 -> [veh_width_buffer_h_Left]
  [Host_Veh_Width] -> Product11/2
  Constant5/1 -> Product11/1
  Delay_Trig/1(Output) -> Hysteresis/1(Input_1)
  Obj_Left_LatDist_Trans/1 -> [ObjsSelect_LatDist_Trans]
  Gain1/1 -> Product1/2
  Gain2/1 -> Add/2
  [Host_Veh_Width] -> Gain4/1
  Gain5/1 -> Product13/2
  Logical Operator5/1 -> Switch/1
  Switch/1 -> [ELKOC_TA_Lateral_OK_Flag_Left]
  Constant/1 -> Switch/2
  [Lateral_Overlap_Left_Flag] -> Switch/3
  Logical Operator6/1 -> [ELKOC_Tar_L_LatRate_OK_Flag]
  Constant19/1 -> First_Order_Lag_Filter/1(filt_loop_time_msec)
  Constant6/1 -> First_Order_Lag_Filter/2(filt_tau_msec)
  Constant17/1 -> First_Order_Lag_Filter1/1(filt_loop_time_msec)
  Constant7/1 -> First_Order_Lag_Filter1/2(filt_tau_msec)
